simulink library "lib_all"  (Simulink 7.6)
settings: Solver=ode45  SolverMode=SingleTasking  StartTime=0.0  StopTime=10.0  RelTol=1e-3
values from neighbouring files (symbols this model uses):
  map_lib_03 = [6.8679 7.0159 7.4571 8.1849 9.1892 10.456 11.97 13.71 15.655 17.781 20.063 22.474 ... (803 elements, 73x11)]    [scr_simdata.mat]    (also in scr_simdata_all.mat)
  map_lib_06 = [2.5784 2.6727 2.9588 3.4453 4.1444 5.068 6.226 7.6242 9.2634 11.139 13.24 15.552 ... (803 elements, 73x11)]    [scr_simdata.mat]    (also in scr_simdata_all.mat)
  map_lib_09 = [0.0025784 0.0026727 0.0029588 0.0034453 0.0041444 0.005068 0.006226 0.0076242 0.0092634 0.011139 0.01324 0.015552 ... (803 elements, 73x11)]    [scr_simdata.mat]    (also in scr_simdata_all.mat)
  map_lib_10 = [2.74 2.75 2.76 2.77 2.78 2.79 2.8 2.81 2.82 2.83 2.84 2.85 ... (81 elements, 1x81)]    [scr_simdata.mat]    (also in scr_simdata_all.mat)
  map_lib_11 = [1.1983 1.2027 1.207 1.2113 1.2156 1.2198 1.2241 1.2283 1.2326 1.2368 1.241 1.2451 ... (81 elements, 1x81)]    [scr_simdata.mat]    (also in scr_simdata_all.mat)
  map_lib_12 = [2.74 2.75 2.76 2.77 2.78 2.79 2.8 2.81 2.82 2.83 2.84 2.85 ... (81 elements, 1x81)]    [scr_simdata.mat]    (also in scr_simdata_all.mat)
  map_lib_13 = [1.74 1.75 1.76 1.77 1.78 1.79 1.8 1.81 1.82 1.83 1.84 1.85 ... (81 elements, 1x81)]    [scr_simdata.mat]    (also in scr_simdata_all.mat)
  map_lib_16 = [0.00048828125 1.6159 1.8962 2.0821 2.2251 2.3426 2.4433 2.5318 2.611 2.683 2.749 2.8101 ... (1419 elements, 129x11)]    [scr_simdata.mat]    (also in scr_simdata_all.mat)
  map_mr2_01 = [0 800 1600 2400 3200 4000]    [scr_simdata.mat]    (also in scr_simdata_all.mat)
  map_mr2_02 = [1.32 1.32 1.33 1.34 1.36 1.38]    [scr_simdata.mat]    (also in scr_simdata_all.mat)
  map_mr2_03 = [0 800 1600 2400 3200 4000]    [scr_simdata.mat]    (also in scr_simdata_all.mat)
  map_mr2_04 = [1.32 1.32 1.33 1.34 1.36 1.38]    [scr_simdata.mat]    (also in scr_simdata_all.mat)
  map_mr2_05 = [0 800 1600 2400 3200 4000]    [scr_simdata.mat]    (also in scr_simdata_all.mat)
  map_mr2_06 = [1.32 1.32 1.33 1.34 1.36 1.38]    [scr_simdata.mat]    (also in scr_simdata_all.mat)
  Parameter1 = 6    [scr_simdata.mat]    (also in scr_simdata_all.mat)
  Parameter101 = 30    [scr_simdata.mat]    (also in scr_simdata_all.mat)
  Parameter102 = 12    [scr_simdata.mat]    (also in scr_simdata_all.mat)
  Parameter103 = -240    [scr_simdata.mat]    (also in scr_simdata_all.mat)
  Parameter108 = 48    [scr_simdata.mat]    (also in scr_simdata_all.mat)
  Parameter120 = 16    [scr_simdata.mat]    (also in scr_simdata_all.mat)
  Parameter121 = 87    [scr_simdata.mat]    (also in scr_simdata_all.mat)
  Parameter123 = 78    [scr_simdata.mat]    (also in scr_simdata_all.mat)
  Parameter124 = 60    [scr_simdata.mat]    (also in scr_simdata_all.mat)
  Parameter125 = -480    [scr_simdata.mat]    (also in scr_simdata_all.mat)
  Parameter130 = 96    [scr_simdata.mat]    (also in scr_simdata_all.mat)
  Parameter131 = 3    [scr_simdata.mat]    (also in scr_simdata_all.mat)
  Parameter144 = 3    [scr_simdata.mat]    (also in scr_simdata_all.mat)
  Parameter18 = 128    [scr_simdata.mat]    (also in scr_simdata_all.mat)
  Parameter19 = 16    [scr_simdata.mat]    (also in scr_simdata_all.mat)
  Parameter2 = 12    [scr_simdata.mat]    (also in scr_simdata_all.mat)
  Parameter20 = 7    [scr_simdata.mat]    (also in scr_simdata_all.mat)
  Parameter21 = 4    [scr_simdata.mat]    (also in scr_simdata_all.mat)
  Parameter3 = 18    [scr_simdata.mat]    (also in scr_simdata_all.mat)
  Parameter32 = 1    [scr_simdata.mat]    (also in scr_simdata_all.mat)
  Parameter34 = 24    [scr_simdata.mat]    (also in scr_simdata_all.mat)
  Parameter4 = 138    [scr_simdata.mat]    (also in scr_simdata_all.mat)
  Parameter42 = 48    [scr_simdata.mat]    (also in scr_simdata_all.mat)
  Parameter5 = 135    [scr_simdata.mat]    (also in scr_simdata_all.mat)
  Parameter55 = 0    [scr_simdata.mat]    (also in scr_simdata_all.mat)
  Parameter56 = 0    [scr_simdata.mat]    (also in scr_simdata_all.mat)
  Parameter57 = 1    [scr_simdata.mat]    (also in scr_simdata_all.mat)
  Parameter58 = 1    [scr_simdata.mat]    (also in scr_simdata_all.mat)
  Parameter59 = 2    [scr_simdata.mat]    (also in scr_simdata_all.mat)
  Parameter6 = 132    [scr_simdata.mat]    (also in scr_simdata_all.mat)
  Parameter60 = 2    [scr_simdata.mat]    (also in scr_simdata_all.mat)
  Parameter63 = 144    [scr_simdata.mat]    (also in scr_simdata_all.mat)
  Parameter7 = 126    [scr_simdata.mat]    (also in scr_simdata_all.mat)
  Parameter76 = 64    [scr_simdata.mat]    (also in scr_simdata_all.mat)
  Parameter77 = 135    [scr_simdata.mat]    (also in scr_simdata_all.mat)
  Parameter79 = 126    [scr_simdata.mat]    (also in scr_simdata_all.mat)
  Parameter80 = 108    [scr_simdata.mat]    (also in scr_simdata_all.mat)
  Parameter81 = 0    [scr_simdata.mat]    (also in scr_simdata_all.mat)
  Parameter86 = 0    [scr_simdata.mat]    (also in scr_simdata_all.mat)
  Parameter98 = 112    [scr_simdata.mat]    (also in scr_simdata_all.mat)
  Parameter99 = 39    [scr_simdata.mat]    (also in scr_simdata_all.mat)
undefined symbols (not defined in the file or in its folder): cylvolkp_hih_map2h, cylvolkp_map2h, pk1k_map2h
libraries: simulink x2
(part 21 of 36)

== system lib_all/SubSystem347/SubSystem349/SubSystem352/SubSystem466/SubSystem469 ==
  Block1042 : Inport
  Block1043 : Inport  Port=2
  Block1044 : ActionPort
  SubSystem470 : Subsystem  TreatAsAtomicUnit=on  mask(***ATC.DSWVEC***){bd_version=1.01.A, r0=DSM392, r1=-1, r2=, r3=, r4=, r5=, r6=, r7=, r8=, r9=, c0=, c1=, c2=, c3=, c4=, c5=, c6=, c7=, c8=, c9=}
      mask prompts: bd_version "Version"
      mask help: これはAutoCからのブロックです．編集禁止．
      settings: OpenFcn=warndlg('変更禁止!');
      mask Help: web(ui_blockhelpmng(gcb));
      mask Display: disp('ATC.DSRVEC')
  wires:
  Block1043/1 -> SubSystem470/2(element)
  Block1042/1 -> SubSystem470/1(input)

== system lib_all/SubSystem347/SubSystem349/SubSystem352/SubSystem466/SubSystem471 ==
  Block1045 : Inport
  Block1046 : Inport  Port=2
  Block1047 : ActionPort
  SubSystem472 : Subsystem  TreatAsAtomicUnit=on  mask(***ATC.DSWVEC***){bd_version=1.01.A, r0=DSM394, r1=-1, r2=, r3=, r4=, r5=, r6=, r7=, r8=, r9=, c0=, c1=, c2=, c3=, c4=, c5=, c6=, c7=, c8=, c9=}
      mask prompts: bd_version "Version"
      mask help: これはAutoCからのブロックです．編集禁止．
      settings: OpenFcn=warndlg('変更禁止!');
      mask Help: web(ui_blockhelpmng(gcb));
      mask Display: disp('ATC.DSRVEC')
  wires:
  Block1046/1 -> SubSystem472/2(element)
  Block1045/1 -> SubSystem472/1(input)

== system lib_all/SubSystem347/SubSystem349/SubSystem352/SubSystem473/SubSystem474 ==
  note: ***編集禁止***
  element : Inport
  Inside_DSR : DataStoreRead  DataStoreName=DSM386
  Selector : Selector  IndexOptions=Index vector (port)  Indices=[1 3]  OutputSizes=1
  Output : Outport
  wires:
  Selector/1 -> Output/1
  element/1 -> Selector/2
  Inside_DSR/1 -> Selector/1

== system lib_all/SubSystem347/SubSystem349/SubSystem352/SubSystem473/SubSystem475 ==
  note: ***編集禁止***
  input : Inport
  element : Inport  Port=2
  Assignment : Assignment  IndexOptions=Index vector (port)  Indices=1  OutputSizes=[1 1]
  Inside_DSR : DataStoreRead  DataStoreName=DSM386
  Inside_DSW : DataStoreWrite  DataStoreName=DSM386
  wires:
  element/1 -> Assignment/3
  input/1 -> Assignment/2
  Inside_DSR/1 -> Assignment/1
  Assignment/1 -> Inside_DSW/1

== system lib_all/SubSystem347/SubSystem477/SubSystem479/SubSystem482/SubSystem483 ==
  note: ***編集禁止***
  In1 : Inport
  In2 : Inport  Port=2
  RO1 : RelationalOperator  Operator=<=  InputSameDT=on
  single1 : DataTypeConversion  OutDataTypeStr=single  SaturateOnIntegerOverflow=on  RndMeth=Zero
  single2 : DataTypeConversion  OutDataTypeStr=single  SaturateOnIntegerOverflow=on  RndMeth=Zero
  Out1 : Outport
  wires:
  RO1/1 -> Out1/1
  In2/1 -> single2/1
  In1/1 -> single1/1
  single1/1 -> RO1/1
  single2/1 -> RO1/2

== system lib_all/SubSystem347/SubSystem477/SubSystem479/SubSystem482/SubSystem484 ==
  note: ***編集禁止***
  input : Inport
  element : Inport  Port=2
  Assignment : Assignment  IndexOptions=Index vector (port)  Indices=1  OutputSizes=[1 1]
  Inside_DSR : DataStoreRead  DataStoreName=DSM383
  Inside_DSW : DataStoreWrite  DataStoreName=DSM383
  wires:
  element/1 -> Assignment/3
  input/1 -> Assignment/2
  Inside_DSR/1 -> Assignment/1
  Assignment/1 -> Inside_DSW/1

== system lib_all/SubSystem347/SubSystem477/SubSystem479/SubSystem482/SubSystem485 ==
  note: ***編集禁止***
  input : Inport
  element : Inport  Port=2
  Assignment : Assignment  IndexOptions=Index vector (port)  Indices=1  OutputSizes=[1 1]
  Inside_DSR : DataStoreRead  DataStoreName=DSM385
  Inside_DSW : DataStoreWrite  DataStoreName=DSM385
  wires:
  element/1 -> Assignment/3
  input/1 -> Assignment/2
  Inside_DSR/1 -> Assignment/1
  Assignment/1 -> Inside_DSW/1

== system lib_all/SubSystem347/SubSystem477/SubSystem479/SubSystem482/SubSystem486 ==
  note: ***編集禁止***
  input : Inport
  element : Inport  Port=2
  Assignment : Assignment  IndexOptions=Index vector (port)  Indices=1  OutputSizes=[1 1]
  Inside_DSR : DataStoreRead  DataStoreName=DSM387
  Inside_DSW : DataStoreWrite  DataStoreName=DSM387
  wires:
  element/1 -> Assignment/3
  input/1 -> Assignment/2
  Inside_DSR/1 -> Assignment/1
  Assignment/1 -> Inside_DSW/1

== system lib_all/SubSystem347/SubSystem477/SubSystem479/SubSystem482/SubSystem487 ==
  Block1085 : EnablePort
  Block1086 : Constant  Value=Parameter131  OutDataTypeStr=double  SampleTime=-1
  Block1087 : Constant  Value=10  OutDataTypeStr=double  SampleTime=-1
  Block1088 : DataStoreWrite  DataStoreName=DSM365
  SubSystem488 : Subsystem  TreatAsAtomicUnit=on
  SubSystem490 : Subsystem  TreatAsAtomicUnit=on
      settings: RTWSystemCode=Inline
  wires:
  Block1087/1 -> Block1088/1
  Block1086/1 -> SubSystem490/1(N)
  SubSystem490/1(action) -> SubSystem488/Trigger
  SubSystem490/2(i) -> SubSystem488/1(Block1089)

== system lib_all/SubSystem347/SubSystem477/SubSystem479/SubSystem492/SubSystem493 ==
  note: ***編集禁止***
  In1 : Inport
  In2 : Inport  Port=2
  RO1 : RelationalOperator  Operator===  InputSameDT=on
  single1 : DataTypeConversion  OutDataTypeStr=single  SaturateOnIntegerOverflow=on  RndMeth=Zero
  single2 : DataTypeConversion  OutDataTypeStr=single  SaturateOnIntegerOverflow=on  RndMeth=Zero
  Out1 : Outport
  wires:
  RO1/1 -> Out1/1
  In2/1 -> single2/1
  In1/1 -> single1/1
  single1/1 -> RO1/1
  single2/1 -> RO1/2

== system lib_all/SubSystem347/SubSystem477/SubSystem479/SubSystem492/SubSystem494 ==
  Block1105 : Inport
  Block1106 : Inport  Port=2
  Block1107 : MultiPortSwitch  zeroidx=off  RndMeth=Zero  SaturateOnIntegerOverflow=on  InputSameDT=on
  SubSystem495 : Subsystem  TreatAsAtomicUnit=on  mask(***ATC.DSRVEC***){bd_version=1.01.A, r0=DSM382, r1=-1, r2=, r3=, r4=, r5=, r6=, r7=, r8=, r9=, c0=, c1=, c2=, c3=, c4=, c5=, c6=, c7=, c8=, c9=}
      mask prompts: bd_version "Version"
      mask help: これはAutoCからのブロックです．編集禁止．
      settings: OpenFcn=warndlg('変更禁止!');
      mask Help: web(ui_blockhelpmng(gcb));
      mask Display: disp('ATC.DSRVEC')
  SubSystem496 : Subsystem  TreatAsAtomicUnit=on  mask(***ATC.DSRVEC***){bd_version=1.01.A, r0=DSM391, r1=-1, r2=, r3=, r4=, r5=, r6=, r7=, r8=, r9=, c0=, c1=, c2=, c3=, c4=, c5=, c6=, c7=, c8=, c9=}
      mask prompts: bd_version "Version"
      mask help: これはAutoCからのブロックです．編集禁止．
      settings: OpenFcn=warndlg('変更禁止!');
      mask Help: web(ui_blockhelpmng(gcb));
      mask Display: disp('ATC.DSRVEC')
  SubSystem497 : Subsystem  TreatAsAtomicUnit=on  mask(***ATC.DSRVEC***){bd_version=1.01.A, r0=DSM393, r1=-1, r2=, r3=, r4=, r5=, r6=, r7=, r8=, r9=, c0=, c1=, c2=, c3=, c4=, c5=, c6=, c7=, c8=, c9=}
      mask prompts: bd_version "Version"
      mask help: これはAutoCからのブロックです．編集禁止．
      settings: OpenFcn=warndlg('変更禁止!');
      mask Help: web(ui_blockhelpmng(gcb));
      mask Display: disp('ATC.DSRVEC')
  Block1108 : Outport
  wires:
  Block1106/1 -> SubSystem497/1(element), SubSystem496/1(element), SubSystem495/1(element)
  Block1107/1 -> Block1108/1
  SubSystem495/1(Output) -> Block1107/2
  SubSystem496/1(Output) -> Block1107/3
  SubSystem497/1(Output) -> Block1107/4
  Block1105/1 -> Block1107/1

== system lib_all/SubSystem347/SubSystem477/SubSystem479/SubSystem492/SubSystem498 ==
  Block1109 : Inport
  Block1110 : Inport  Port=2
  Block1111 : MultiPortSwitch  zeroidx=off  RndMeth=Zero  SaturateOnIntegerOverflow=on  InputSameDT=on
  SubSystem499 : Subsystem  TreatAsAtomicUnit=on  mask(***ATC.DSRVEC***){bd_version=1.01.A, r0=DSM384, r1=-1, r2=, r3=, r4=, r5=, r6=, r7=, r8=, r9=, c0=, c1=, c2=, c3=, c4=, c5=, c6=, c7=, c8=, c9=}
      mask prompts: bd_version "Version"
      mask help: これはAutoCからのブロックです．編集禁止．
      settings: OpenFcn=warndlg('変更禁止!');
      mask Help: web(ui_blockhelpmng(gcb));
      mask Display: disp('ATC.DSRVEC')
  SubSystem500 : Subsystem  TreatAsAtomicUnit=on  mask(***ATC.DSRVEC***){bd_version=1.01.A, r0=DSM392, r1=-1, r2=, r3=, r4=, r5=, r6=, r7=, r8=, r9=, c0=, c1=, c2=, c3=, c4=, c5=, c6=, c7=, c8=, c9=}
      mask prompts: bd_version "Version"
      mask help: これはAutoCからのブロックです．編集禁止．
      settings: OpenFcn=warndlg('変更禁止!');
      mask Help: web(ui_blockhelpmng(gcb));
      mask Display: disp('ATC.DSRVEC')
  SubSystem501 : Subsystem  TreatAsAtomicUnit=on  mask(***ATC.DSRVEC***){bd_version=1.01.A, r0=DSM394, r1=-1, r2=, r3=, r4=, r5=, r6=, r7=, r8=, r9=, c0=, c1=, c2=, c3=, c4=, c5=, c6=, c7=, c8=, c9=}
      mask prompts: bd_version "Version"
      mask help: これはAutoCからのブロックです．編集禁止．
      settings: OpenFcn=warndlg('変更禁止!');
      mask Help: web(ui_blockhelpmng(gcb));
      mask Display: disp('ATC.DSRVEC')
  Block1112 : Outport
  wires:
  Block1110/1 -> SubSystem501/1(element), SubSystem500/1(element), SubSystem499/1(element)
  SubSystem501/1(Output) -> Block1111/4
  SubSystem500/1(Output) -> Block1111/3
  SubSystem499/1(Output) -> Block1111/2
  Block1111/1 -> Block1112/1
  Block1109/1 -> Block1111/1

== system lib_all/SubSystem347/SubSystem503/SubSystem506/SubSystem511/SubSystem512 ==
  note: ***編集禁止***
  input : Inport
  element : Inport  Port=2
  Assignment : Assignment  IndexOptions=Index vector (port)  Indices=1  OutputSizes=[1 1]
  Inside_DSR : DataStoreRead  DataStoreName=DSM87
  Inside_DSW : DataStoreWrite  DataStoreName=DSM87
  wires:
  element/1 -> Assignment/3
  input/1 -> Assignment/2
  Inside_DSR/1 -> Assignment/1
  Assignment/1 -> Inside_DSW/1

== system lib_all/SubSystem347/SubSystem503/SubSystem506/SubSystem511/SubSystem513 ==
  note: ***編集禁止***
  element : Inport
  Inside_DSR : DataStoreRead  DataStoreName=DSM387
  Selector : Selector  IndexOptions=Index vector (port)  Indices=[1 3]  OutputSizes=1
  Output : Outport
  wires:
  Selector/1 -> Output/1
  element/1 -> Selector/2
  Inside_DSR/1 -> Selector/1

== system lib_all/SubSystem347/SubSystem503/SubSystem506/SubSystem511/SubSystem514 ==
  note: ***編集禁止***
  element : Inport
  Inside_DSR : DataStoreRead  DataStoreName=DSM387
  Selector : Selector  IndexOptions=Index vector (port)  Indices=[1 3]  OutputSizes=1
  Output : Outport
  wires:
  Selector/1 -> Output/1
  element/1 -> Selector/2
  Inside_DSR/1 -> Selector/1

== system lib_all/SubSystem347/SubSystem503/SubSystem506/SubSystem515/SubSystem516 ==
  note: ***編集禁止***
  input : Inport
  element : Inport  Port=2
  Assignment : Assignment  IndexOptions=Index vector (port)  Indices=1  OutputSizes=[1 1]
  Inside_DSR : DataStoreRead  DataStoreName=DSM386
  Inside_DSW : DataStoreWrite  DataStoreName=DSM386
  wires:
  element/1 -> Assignment/3
  input/1 -> Assignment/2
  Inside_DSR/1 -> Assignment/1
  Assignment/1 -> Inside_DSW/1

== system lib_all/SubSystem518/SubSystem523/SubSystem524/SubSystem527/SubSystem528 ==
  note: ***編集禁止***
  mult : Inport
  div : Inport  Port=2
  init : Inport  Port=3
  Product : Subsystem  TreatAsAtomicUnit=on
      settings: RTWSystemCode=Inline
  Out : Outport
  wires:
  init/1 -> Product/3(init)
  div/1 -> Product/2(div)
  mult/1 -> Product/1(mult)
  Product/1(Out) -> Out/1

== system lib_all/SubSystem518/SubSystem523/SubSystem524/SubSystem527/SubSystem529 ==
  note: ***編集禁止***
  element : Inport
  Inside_DSR : DataStoreRead  DataStoreName=DSM95
  Selector : Selector  IndexOptions=Index vector (port)  Indices=[1 3]  OutputSizes=1
  Output : Outport
  wires:
  Inside_DSR/1 -> Selector/1
  element/1 -> Selector/2
  Selector/1 -> Output/1

== system lib_all/SubSystem518/SubSystem523/SubSystem524/SubSystem527/SubSystem530 ==
  Block1193 : Inport
  Block1194 : Inport  Port=2
  Block1195 : Inport  Port=3
  Block1196 : Sum  OutDataTypeStr=double  RndMeth=Zero  SaturateOnIntegerOverflow=on  InputSameDT=on
  Block1197 : Sum  Inputs=+-  OutDataTypeStr=double  RndMeth=Zero  SaturateOnIntegerOverflow=on  InputSameDT=on
  Block1198 : Switch  Threshold=0.5  RndMeth=Zero  SaturateOnIntegerOverflow=on  InputSameDT=on
  Block1199 : Switch  Threshold=0.5  RndMeth=Zero  SaturateOnIntegerOverflow=on  InputSameDT=on
  SubSystem531 : Subsystem
  SubSystem532 : Subsystem
  Block1200 : Outport
  wires:
  Block1197/1 -> Block1198/1
  Block1198/1 -> Block1200/1
  Block1196/1 -> Block1199/1
  Block1199/1 -> Block1198/3
  SubSystem532/1(Out1) -> Block1199/2
  Block1195/1 -> SubSystem532/2(In2)
  SubSystem531/1(Out1) -> Block1198/2
  Block1193/1 -> SubSystem531/2(In2), Block1197/2, Block1196/1
  Block1194/1 -> Block1196/2, Block1199/3, SubSystem532/1(In1), Block1197/1, SubSystem531/1(In1)

== system lib_all/SubSystem518/SubSystem523/SubSystem524/SubSystem533/SubSystem534 ==
  note: ***編集禁止***
  In1 : Inport
  In2 : Inport  Port=2
  LO1 : Logic
  RO1 : RelationalOperator  Operator=<  InputSameDT=on
  RO2 : RelationalOperator  Operator=~=  InputSameDT=on
  single1 : DataTypeConversion  OutDataTypeStr=single  SaturateOnIntegerOverflow=on  RndMeth=Zero
  single2 : DataTypeConversion  OutDataTypeStr=single  SaturateOnIntegerOverflow=on  RndMeth=Zero
  Out1 : Outport
  wires:
  single2/1 -> RO1/2, RO2/2
  single1/1 -> RO1/1, RO2/1
  In1/1 -> single1/1
  In2/1 -> single2/1
  RO1/1 -> LO1/1
  RO2/1 -> LO1/2
  LO1/1 -> Out1/1

== system lib_all/SubSystem518/SubSystem523/SubSystem524/SubSystem533/SubSystem535 ==
  note: ***編集禁止***
  In1 : Inport
  In2 : Inport  Port=2
  RO1 : RelationalOperator  Operator===  InputSameDT=on
  single1 : DataTypeConversion  OutDataTypeStr=single  SaturateOnIntegerOverflow=on  RndMeth=Zero
  single2 : DataTypeConversion  OutDataTypeStr=single  SaturateOnIntegerOverflow=on  RndMeth=Zero
  Out1 : Outport
  wires:
  single2/1 -> RO1/2
  single1/1 -> RO1/1
  In1/1 -> single1/1
  In2/1 -> single2/1
  RO1/1 -> Out1/1

== system lib_all/SubSystem518/SubSystem523/SubSystem524/SubSystem533/SubSystem536 ==
  note: ***編集禁止***
  In1 : Inport
  In2 : Inport  Port=2
  RO1 : RelationalOperator  Operator===  InputSameDT=on
  single1 : DataTypeConversion  OutDataTypeStr=single  SaturateOnIntegerOverflow=on  RndMeth=Zero
  single2 : DataTypeConversion  OutDataTypeStr=single  SaturateOnIntegerOverflow=on  RndMeth=Zero
  Out1 : Outport
  wires:
  single2/1 -> RO1/2
  single1/1 -> RO1/1
  In1/1 -> single1/1
  In2/1 -> single2/1
  RO1/1 -> Out1/1

== system lib_all/SubSystem518/SubSystem523/SubSystem524/SubSystem533/SubSystem537 ==
  note: ***編集禁止***
  In1 : Inport
  In2 : Inport  Port=2
  RO1 : RelationalOperator  Operator===  InputSameDT=on
  single1 : DataTypeConversion  OutDataTypeStr=single  SaturateOnIntegerOverflow=on  RndMeth=Zero
  single2 : DataTypeConversion  OutDataTypeStr=single  SaturateOnIntegerOverflow=on  RndMeth=Zero
  Out1 : Outport
  wires:
  single2/1 -> RO1/2
  single1/1 -> RO1/1
  In1/1 -> single1/1
  In2/1 -> single2/1
  RO1/1 -> Out1/1

== system lib_all/SubSystem518/SubSystem523/SubSystem524/SubSystem533/SubSystem538 ==
  Block1223 : Inport
  Block1224 : Inport  Port=2
  Block1225 : EnablePort
  Block1226 : Demux  Outputs=2
  Block1227 : Mux  Inputs=2
  SubSystem539 : Subsystem
  SubSystem543 : Subsystem
  Block1236 : Outport
  wires:
  Block1227/1 -> Block1236/1
  SubSystem543/1(Block1235) -> Block1227/2
  SubSystem539/1(Block1231) -> Block1227/1
  Block1226/2 -> SubSystem543/2(Block1233)
  Block1226/1 -> SubSystem539/2(Block1229)
  Block1224/1 -> SubSystem539/1(Block1228), SubSystem543/1(Block1232)
  Block1223/1 -> Block1226/1

== system lib_all/SubSystem518/SubSystem523/SubSystem524/SubSystem533/SubSystem547 ==
  Block1237 : Inport
  Block1238 : Inport  Port=2
  Block1239 : EnablePort
  Block1240 : Demux  Outputs=2
  Block1241 : Mux  Inputs=2
  Block1242 : Sum  OutDataTypeStr=double  RndMeth=Zero  SaturateOnIntegerOverflow=on  InputSameDT=on
  SubSystem548 : Subsystem  TreatAsAtomicUnit=on  mask(***ATC.DSRVEC***){bd_version=1.01.A, r0=DSM1, r1=-1, r2=, r3=, r4=, r5=, r6=, r7=, r8=, r9=, c0=, c1=, c2=, c3=, c4=, c5=, c6=, c7=, c8=, c9=}
      mask prompts: bd_version "Version"
      mask help: これはAutoCからのブロックです．編集禁止．
      settings: OpenFcn=warndlg('変更禁止!');
      mask Help: web(ui_blockhelpmng(gcb));
      mask Display: disp('ATC.DSRVEC')
  SubSystem549 : Subsystem
  SubSystem553 : Subsystem
  Block1251 : Outport
  wires:
  Block1238/1 -> SubSystem548/1(element), SubSystem549/1(Block1243), SubSystem553/1(Block1247)
  Block1241/1 -> Block1242/2
  SubSystem548/1(Output) -> Block1242/1
  Block1242/1 -> Block1251/1
  SubSystem553/1(Block1250) -> Block1241/2
  SubSystem549/1(Block1246) -> Block1241/1
  Block1240/2 -> SubSystem553/2(Block1248)
  Block1240/1 -> SubSystem549/2(Block1244)
  Block1237/1 -> Block1240/1

== system lib_all/SubSystem518/SubSystem523/SubSystem524/SubSystem533/SubSystem557 ==
  Block1252 : Inport
  Block1253 : Inport  Port=2
  Block1254 : EnablePort
  Block1255 : Demux  Outputs=2
  Block1256 : Mux  Inputs=2
  Block1257 : Sum  OutDataTypeStr=double  RndMeth=Zero  SaturateOnIntegerOverflow=on  InputSameDT=on
  SubSystem558 : Subsystem  TreatAsAtomicUnit=on  mask(***ATC.DSRVEC***){bd_version=1.01.A, r0=DSM5, r1=-1, r2=, r3=, r4=, r5=, r6=, r7=, r8=, r9=, c0=, c1=, c2=, c3=, c4=, c5=, c6=, c7=, c8=, c9=}
      mask prompts: bd_version "Version"
      mask help: これはAutoCからのブロックです．編集禁止．
      settings: OpenFcn=warndlg('変更禁止!');
      mask Help: web(ui_blockhelpmng(gcb));
      mask Display: disp('ATC.DSRVEC')
  SubSystem559 : Subsystem
  SubSystem563 : Subsystem
  Block1266 : Outport
  wires:
  Block1253/1 -> SubSystem558/1(element), SubSystem559/1(Block1258), SubSystem563/1(Block1262)
  Block1256/1 -> Block1257/2
  SubSystem558/1(Output) -> Block1257/1
  Block1257/1 -> Block1266/1
  SubSystem563/1(Block1265) -> Block1256/2
  SubSystem559/1(Block1261) -> Block1256/1
  Block1255/2 -> SubSystem563/2(Block1263)
  Block1255/1 -> SubSystem559/2(Block1259)
  Block1252/1 -> Block1255/1

== system lib_all/SubSystem518/SubSystem523/SubSystem524/SubSystem533/SubSystem567 ==
  Block1267 : Inport
  Block1268 : Inport  Port=2
  Block1269 : EnablePort
  Block1270 : Demux  Outputs=2
  Block1271 : Mux  Inputs=2
  Block1272 : Sum  OutDataTypeStr=double  RndMeth=Zero  SaturateOnIntegerOverflow=on  InputSameDT=on
  SubSystem568 : Subsystem  TreatAsAtomicUnit=on  mask(***ATC.DSRVEC***){bd_version=1.01.A, r0=DSM3, r1=-1, r2=, r3=, r4=, r5=, r6=, r7=, r8=, r9=, c0=, c1=, c2=, c3=, c4=, c5=, c6=, c7=, c8=, c9=}
      mask prompts: bd_version "Version"
      mask help: これはAutoCからのブロックです．編集禁止．
      settings: OpenFcn=warndlg('変更禁止!');
      mask Help: web(ui_blockhelpmng(gcb));
      mask Display: disp('ATC.DSRVEC')
  SubSystem569 : Subsystem
  SubSystem573 : Subsystem
  Block1281 : Outport
  wires:
  Block1268/1 -> SubSystem568/1(element), SubSystem569/1(Block1273), SubSystem573/1(Block1277)
  Block1267/1 -> Block1270/1
  Block1270/1 -> SubSystem569/2(Block1274)
  Block1270/2 -> SubSystem573/2(Block1278)
  SubSystem569/1(Block1276) -> Block1271/1
  SubSystem573/1(Block1280) -> Block1271/2
  Block1272/1 -> Block1281/1
  SubSystem568/1(Output) -> Block1272/1
  Block1271/1 -> Block1272/2

== system lib_all/SubSystem518/SubSystem523/SubSystem577/SubSystem580/SubSystem581 ==
  note: ***編集禁止***
  mult : Inport
  div : Inport  Port=2
  init : Inport  Port=3
  Product : Subsystem  TreatAsAtomicUnit=on
      settings: RTWSystemCode=Inline
  Out : Outport
  wires:
  init/1 -> Product/3(init)
  div/1 -> Product/2(div)
  mult/1 -> Product/1(mult)
  Product/1(Out) -> Out/1

== system lib_all/SubSystem518/SubSystem523/SubSystem577/SubSystem580/SubSystem582 ==
  note: ***編集禁止***
  element : Inport
  Inside_DSR : DataStoreRead  DataStoreName=DSM95
  Selector : Selector  IndexOptions=Index vector (port)  Indices=[1 3]  OutputSizes=1
  Output : Outport
  wires:
  Inside_DSR/1 -> Selector/1
  element/1 -> Selector/2
  Selector/1 -> Output/1

== system lib_all/SubSystem518/SubSystem523/SubSystem577/SubSystem580/SubSystem583 ==
  Block1315 : Inport
  Block1316 : Inport  Port=2
  Block1317 : Inport  Port=3
  Block1318 : Sum  OutDataTypeStr=double  RndMeth=Zero  SaturateOnIntegerOverflow=on  InputSameDT=on
  Block1319 : Sum  Inputs=+-  OutDataTypeStr=double  RndMeth=Zero  SaturateOnIntegerOverflow=on  InputSameDT=on
  Block1320 : Switch  Threshold=0.5  RndMeth=Zero  SaturateOnIntegerOverflow=on  InputSameDT=on
  Block1321 : Switch  Threshold=0.5  RndMeth=Zero  SaturateOnIntegerOverflow=on  InputSameDT=on
  SubSystem584 : Subsystem
  SubSystem585 : Subsystem
  Block1322 : Outport
  wires:
  Block1319/1 -> Block1320/1
  Block1320/1 -> Block1322/1
  Block1318/1 -> Block1321/1
  Block1321/1 -> Block1320/3
  SubSystem585/1(Out1) -> Block1321/2
  Block1317/1 -> SubSystem585/2(In2)
  SubSystem584/1(Out1) -> Block1320/2
  Block1315/1 -> SubSystem584/2(In2), Block1319/2, Block1318/1
  Block1316/1 -> Block1318/2, Block1321/3, SubSystem585/1(In1), Block1319/1, SubSystem584/1(In1)

== system lib_all/SubSystem518/SubSystem523/SubSystem577/SubSystem586/SubSystem587 ==
  note: ***編集禁止***
  In1 : Inport
  In2 : Inport  Port=2
  LO1 : Logic
  RO1 : RelationalOperator  Operator=<  InputSameDT=on
  RO2 : RelationalOperator  Operator=~=  InputSameDT=on
  single1 : DataTypeConversion  OutDataTypeStr=single  SaturateOnIntegerOverflow=on  RndMeth=Zero
  single2 : DataTypeConversion  OutDataTypeStr=single  SaturateOnIntegerOverflow=on  RndMeth=Zero
  Out1 : Outport
  wires:
  single2/1 -> RO1/2, RO2/2
  single1/1 -> RO1/1, RO2/1
  In1/1 -> single1/1
  In2/1 -> single2/1
  RO1/1 -> LO1/1
  RO2/1 -> LO1/2
  LO1/1 -> Out1/1

== system lib_all/SubSystem518/SubSystem523/SubSystem577/SubSystem586/SubSystem588 ==
  note: ***編集禁止***
  In1 : Inport
  In2 : Inport  Port=2
  RO1 : RelationalOperator  Operator===  InputSameDT=on
  single1 : DataTypeConversion  OutDataTypeStr=single  SaturateOnIntegerOverflow=on  RndMeth=Zero
  single2 : DataTypeConversion  OutDataTypeStr=single  SaturateOnIntegerOverflow=on  RndMeth=Zero
  Out1 : Outport
  wires:
  single2/1 -> RO1/2
  single1/1 -> RO1/1
  In1/1 -> single1/1
  In2/1 -> single2/1
  RO1/1 -> Out1/1

== system lib_all/SubSystem518/SubSystem523/SubSystem577/SubSystem586/SubSystem589 ==
  note: ***編集禁止***
  In1 : Inport
  In2 : Inport  Port=2
  RO1 : RelationalOperator  Operator===  InputSameDT=on
  single1 : DataTypeConversion  OutDataTypeStr=single  SaturateOnIntegerOverflow=on  RndMeth=Zero
  single2 : DataTypeConversion  OutDataTypeStr=single  SaturateOnIntegerOverflow=on  RndMeth=Zero
  Out1 : Outport
  wires:
  single2/1 -> RO1/2
  single1/1 -> RO1/1
  In1/1 -> single1/1
  In2/1 -> single2/1
  RO1/1 -> Out1/1

== system lib_all/SubSystem518/SubSystem523/SubSystem577/SubSystem586/SubSystem590 ==
  note: ***編集禁止***
  In1 : Inport
  In2 : Inport  Port=2
  RO1 : RelationalOperator  Operator===  InputSameDT=on
  single1 : DataTypeConversion  OutDataTypeStr=single  SaturateOnIntegerOverflow=on  RndMeth=Zero
  single2 : DataTypeConversion  OutDataTypeStr=single  SaturateOnIntegerOverflow=on  RndMeth=Zero
  Out1 : Outport
  wires:
  single2/1 -> RO1/2
  single1/1 -> RO1/1
  In1/1 -> single1/1
  In2/1 -> single2/1
  RO1/1 -> Out1/1

== system lib_all/SubSystem518/SubSystem523/SubSystem577/SubSystem586/SubSystem591 ==
  Block1345 : Inport
  Block1346 : Inport  Port=2
  Block1347 : EnablePort
  Block1348 : Demux  Outputs=2
  Block1349 : Mux  Inputs=2
  SubSystem592 : Subsystem
  SubSystem596 : Subsystem
  Block1358 : Outport
  wires:
  Block1349/1 -> Block1358/1
  SubSystem596/1(Block1357) -> Block1349/2
  SubSystem592/1(Block1353) -> Block1349/1
  Block1348/2 -> SubSystem596/2(Block1355)
  Block1348/1 -> SubSystem592/2(Block1351)
  Block1346/1 -> SubSystem592/1(Block1350), SubSystem596/1(Block1354)
  Block1345/1 -> Block1348/1

== system lib_all/SubSystem518/SubSystem523/SubSystem577/SubSystem586/SubSystem600 ==
  Block1359 : Inport
  Block1360 : Inport  Port=2
  Block1361 : EnablePort
  Block1362 : Demux  Outputs=2
  Block1363 : Mux  Inputs=2
  Block1364 : Sum  OutDataTypeStr=double  RndMeth=Zero  SaturateOnIntegerOverflow=on  InputSameDT=on
  SubSystem601 : Subsystem  TreatAsAtomicUnit=on  mask(***ATC.DSRVEC***){bd_version=1.01.A, r0=DSM1, r1=-1, r2=, r3=, r4=, r5=, r6=, r7=, r8=, r9=, c0=, c1=, c2=, c3=, c4=, c5=, c6=, c7=, c8=, c9=}
      mask prompts: bd_version "Version"
      mask help: これはAutoCからのブロックです．編集禁止．
      settings: OpenFcn=warndlg('変更禁止!');
      mask Help: web(ui_blockhelpmng(gcb));
      mask Display: disp('ATC.DSRVEC')
  SubSystem602 : Subsystem
  SubSystem606 : Subsystem
  Block1373 : Outport
  wires:
  Block1360/1 -> SubSystem601/1(element), SubSystem602/1(Block1365), SubSystem606/1(Block1369)
  Block1363/1 -> Block1364/2
  SubSystem601/1(Output) -> Block1364/1
  Block1364/1 -> Block1373/1
  SubSystem606/1(Block1372) -> Block1363/2
  SubSystem602/1(Block1368) -> Block1363/1
  Block1362/2 -> SubSystem606/2(Block1370)
  Block1362/1 -> SubSystem602/2(Block1366)
  Block1359/1 -> Block1362/1

== system lib_all/SubSystem518/SubSystem523/SubSystem577/SubSystem586/SubSystem610 ==
  Block1374 : Inport
  Block1375 : Inport  Port=2
  Block1376 : EnablePort
  Block1377 : Demux  Outputs=2
  Block1378 : Mux  Inputs=2
  Block1379 : Sum  OutDataTypeStr=double  RndMeth=Zero  SaturateOnIntegerOverflow=on  InputSameDT=on
  SubSystem611 : Subsystem  TreatAsAtomicUnit=on  mask(***ATC.DSRVEC***){bd_version=1.01.A, r0=DSM5, r1=-1, r2=, r3=, r4=, r5=, r6=, r7=, r8=, r9=, c0=, c1=, c2=, c3=, c4=, c5=, c6=, c7=, c8=, c9=}
      mask prompts: bd_version "Version"
      mask help: これはAutoCからのブロックです．編集禁止．
      settings: OpenFcn=warndlg('変更禁止!');
      mask Help: web(ui_blockhelpmng(gcb));
      mask Display: disp('ATC.DSRVEC')
  SubSystem612 : Subsystem
  SubSystem616 : Subsystem
  Block1388 : Outport
  wires:
  Block1375/1 -> SubSystem611/1(element), SubSystem612/1(Block1380), SubSystem616/1(Block1384)
  Block1378/1 -> Block1379/2
  SubSystem611/1(Output) -> Block1379/1
  Block1379/1 -> Block1388/1
  SubSystem616/1(Block1387) -> Block1378/2
  SubSystem612/1(Block1383) -> Block1378/1
  Block1377/2 -> SubSystem616/2(Block1385)
  Block1377/1 -> SubSystem612/2(Block1381)
  Block1374/1 -> Block1377/1

== system lib_all/SubSystem518/SubSystem523/SubSystem577/SubSystem586/SubSystem620 ==
  Block1389 : Inport
  Block1390 : Inport  Port=2
  Block1391 : EnablePort
  Block1392 : Demux  Outputs=2
  Block1393 : Mux  Inputs=2
  Block1394 : Sum  OutDataTypeStr=double  RndMeth=Zero  SaturateOnIntegerOverflow=on  InputSameDT=on
  SubSystem621 : Subsystem  TreatAsAtomicUnit=on  mask(***ATC.DSRVEC***){bd_version=1.01.A, r0=DSM3, r1=-1, r2=, r3=, r4=, r5=, r6=, r7=, r8=, r9=, c0=, c1=, c2=, c3=, c4=, c5=, c6=, c7=, c8=, c9=}
      mask prompts: bd_version "Version"
      mask help: これはAutoCからのブロックです．編集禁止．
      settings: OpenFcn=warndlg('変更禁止!');
      mask Help: web(ui_blockhelpmng(gcb));
      mask Display: disp('ATC.DSRVEC')
  SubSystem622 : Subsystem
  SubSystem626 : Subsystem
  Block1403 : Outport
  wires:
  Block1390/1 -> SubSystem621/1(element), SubSystem622/1(Block1395), SubSystem626/1(Block1399)
  Block1389/1 -> Block1392/1
  Block1392/1 -> SubSystem622/2(Block1396)
  Block1392/2 -> SubSystem626/2(Block1400)
  SubSystem622/1(Block1398) -> Block1393/1
  SubSystem626/1(Block1402) -> Block1393/2
  Block1394/1 -> Block1403/1
  SubSystem621/1(Output) -> Block1394/1
  Block1393/1 -> Block1394/2

== system lib_all/SubSystem660/SubSystem673/SubSystem676/SubSystem679/SubSystem680 ==
  note: ***編集禁止***
  In1 : Inport
  In2 : Inport  Port=2
  LO1 : Logic
  RO1 : RelationalOperator  Operator=<  InputSameDT=on
  RO2 : RelationalOperator  Operator=~=  InputSameDT=on
  single1 : DataTypeConversion  OutDataTypeStr=single  SaturateOnIntegerOverflow=on  RndMeth=Zero
  single2 : DataTypeConversion  OutDataTypeStr=single  SaturateOnIntegerOverflow=on  RndMeth=Zero
  Out1 : Outport
  wires:
  LO1/1 -> Out1/1
  RO2/1 -> LO1/2
  RO1/1 -> LO1/1
  single2/1 -> RO2/2, RO1/2
  single1/1 -> RO2/1, RO1/1
  In1/1 -> single1/1
  In2/1 -> single2/1

== system lib_all/SubSystem660/SubSystem673/SubSystem676/SubSystem681/SubSystem682 ==
  note: ***編集禁止***
  In1 : Inport
  In2 : Inport  Port=2
  LO1 : Logic
  RO1 : RelationalOperator  Operator=<  InputSameDT=on
  RO2 : RelationalOperator  Operator=~=  InputSameDT=on
  single1 : DataTypeConversion  OutDataTypeStr=single  SaturateOnIntegerOverflow=on  RndMeth=Zero
  single2 : DataTypeConversion  OutDataTypeStr=single  SaturateOnIntegerOverflow=on  RndMeth=Zero
  Out1 : Outport
  wires:
  LO1/1 -> Out1/1
  RO2/1 -> LO1/2
  RO1/1 -> LO1/1
  single2/1 -> RO2/2, RO1/2
  single1/1 -> RO2/1, RO1/1
  In1/1 -> single1/1
  In2/1 -> single2/1

== system lib_all/SubSystem660/SubSystem686/SubSystem688/SubSystem689/Product ==
  note: ***編集禁止***
  mult : Inport
  div : Inport  Port=2
  init : Inport  Port=3
  Constant1 : Constant  OutDataTypeStr=Inherit: Inherit via back propagation
  Constant2 : Constant  Value=0  OutDataTypeStr=Inherit: Inherit via back propagation
  Product : Product  Inputs=*/  SaturateOnIntegerOverflow=on  RndMeth=Zero  InputSameDT=on  OutDataTypeStr=Inherit: Same as first input
  Relational Operator : RelationalOperator  Operator=~=  InputSameDT=on
  Switch : Switch  Threshold=0.5  SaturateOnIntegerOverflow=on  InputSameDT=on
  Switch1 : Switch  Threshold=0.5  SaturateOnIntegerOverflow=on  InputSameDT=on
  Out : Outport
  wires:
  mult/1 -> Product/1
  Product/1 -> Switch/1
  Relational Operator/1 -> Switch/2, Switch1/2
  init/1 -> Switch/3
  Switch/1 -> Out/1
  div/1 -> Switch1/1, Relational Operator/1
  Switch1/1 -> Product/2
  Constant2/1 -> Relational Operator/2
  Constant1/1 -> Switch1/3

== system lib_all/SubSystem660/SubSystem686/SubSystem688/SubSystem691/SubSystem692 ==
  note: ***編集禁止***
  In1 : Inport
  In2 : Inport  Port=2
  RO1 : RelationalOperator  Operator=>  InputSameDT=on
  single1 : DataTypeConversion  OutDataTypeStr=single  SaturateOnIntegerOverflow=on  RndMeth=Zero
  single2 : DataTypeConversion  OutDataTypeStr=single  SaturateOnIntegerOverflow=on  RndMeth=Zero
  Out1 : Outport
  wires:
  RO1/1 -> Out1/1
  In2/1 -> single2/1
  In1/1 -> single1/1
  single1/1 -> RO1/1
  single2/1 -> RO1/2

== system lib_all/SubSystem660/SubSystem686/SubSystem688/SubSystem691/SubSystem693 ==
  note: ***編集禁止***
  In1 : Inport
  In2 : Inport  Port=2
  LO1 : Logic
  RO1 : RelationalOperator  Operator=<  InputSameDT=on
  RO2 : RelationalOperator  Operator=~=  InputSameDT=on
  single1 : DataTypeConversion  OutDataTypeStr=single  SaturateOnIntegerOverflow=on  RndMeth=Zero
  single2 : DataTypeConversion  OutDataTypeStr=single  SaturateOnIntegerOverflow=on  RndMeth=Zero
  Out1 : Outport
  wires:
  In2/1 -> single2/1
  In1/1 -> single1/1
  single1/1 -> RO1/1, RO2/1
  single2/1 -> RO1/2, RO2/2
  RO1/1 -> LO1/1
  RO2/1 -> LO1/2
  LO1/1 -> Out1/1

== system lib_all/SubSystem660/SubSystem686/SubSystem694/SubSystem698/SubSystem699 ==
  Block1612 : Inport
  Block1613 : Inport  Port=2
  Block1614 : MultiPortSwitch  zeroidx=off  RndMeth=Zero  SaturateOnIntegerOverflow=on  InputSameDT=on
  SubSystem700 : Subsystem  TreatAsAtomicUnit=on  mask(***ATC.DSRVEC***){bd_version=1.01.A, r0=DSM18, r1=-1, r2=, r3=, r4=, r5=, r6=, r7=, r8=, r9=, c0=, c1=, c2=, c3=, c4=, c5=, c6=, c7=, c8=, c9=}
      mask prompts: bd_version "Version"
      mask help: これはAutoCからのブロックです．編集禁止．
      settings: OpenFcn=warndlg('変更禁止!');
      mask Help: web(ui_blockhelpmng(gcb));
      mask Display: disp('ATC.DSRVEC')
  SubSystem701 : Subsystem  TreatAsAtomicUnit=on  mask(***ATC.DSRVEC***){bd_version=1.01.A, r0=DSM8, r1=-1, r2=, r3=, r4=, r5=, r6=, r7=, r8=, r9=, c0=, c1=, c2=, c3=, c4=, c5=, c6=, c7=, c8=, c9=}
      mask prompts: bd_version "Version"
      mask help: これはAutoCからのブロックです．編集禁止．
      settings: OpenFcn=warndlg('変更禁止!');
      mask Help: web(ui_blockhelpmng(gcb));
      mask Display: disp('ATC.DSRVEC')
  SubSystem702 : Subsystem  TreatAsAtomicUnit=on  mask(***ATC.DSRVEC***){bd_version=1.01.A, r0=DSM11, r1=-1, r2=, r3=, r4=, r5=, r6=, r7=, r8=, r9=, c0=, c1=, c2=, c3=, c4=, c5=, c6=, c7=, c8=, c9=}
      mask prompts: bd_version "Version"
      mask help: これはAutoCからのブロックです．編集禁止．
      settings: OpenFcn=warndlg('変更禁止!');
      mask Help: web(ui_blockhelpmng(gcb));
      mask Display: disp('ATC.DSRVEC')
  Block1615 : Outport
  wires:
  Block1613/1 -> SubSystem702/1(element), SubSystem701/1(element), SubSystem700/1(element)
  SubSystem702/1(Output) -> Block1614/4
  SubSystem701/1(Output) -> Block1614/3
  SubSystem700/1(Output) -> Block1614/2
  Block1614/1 -> Block1615/1
  Block1612/1 -> Block1614/1

== system lib_all/SubSystem660/SubSystem686/SubSystem694/SubSystem698/SubSystem703 ==
  Block1616 : Inport
  Block1617 : Inport  Port=2
  Block1618 : MultiPortSwitch  zeroidx=off  RndMeth=Zero  SaturateOnIntegerOverflow=on  InputSameDT=on
  SubSystem704 : Subsystem  TreatAsAtomicUnit=on  mask(***ATC.DSRVEC***){bd_version=1.01.A, r0=DSM18, r1=-1, r2=, r3=, r4=, r5=, r6=, r7=, r8=, r9=, c0=, c1=, c2=, c3=, c4=, c5=, c6=, c7=, c8=, c9=}
      mask prompts: bd_version "Version"
      mask help: これはAutoCからのブロックです．編集禁止．
      settings: OpenFcn=warndlg('変更禁止!');
      mask Help: web(ui_blockhelpmng(gcb));
      mask Display: disp('ATC.DSRVEC')
  SubSystem705 : Subsystem  TreatAsAtomicUnit=on  mask(***ATC.DSRVEC***){bd_version=1.01.A, r0=DSM8, r1=-1, r2=, r3=, r4=, r5=, r6=, r7=, r8=, r9=, c0=, c1=, c2=, c3=, c4=, c5=, c6=, c7=, c8=, c9=}
      mask prompts: bd_version "Version"
      mask help: これはAutoCからのブロックです．編集禁止．
      settings: OpenFcn=warndlg('変更禁止!');
      mask Help: web(ui_blockhelpmng(gcb));
      mask Display: disp('ATC.DSRVEC')
  SubSystem706 : Subsystem  TreatAsAtomicUnit=on  mask(***ATC.DSRVEC***){bd_version=1.01.A, r0=DSM11, r1=-1, r2=, r3=, r4=, r5=, r6=, r7=, r8=, r9=, c0=, c1=, c2=, c3=, c4=, c5=, c6=, c7=, c8=, c9=}
      mask prompts: bd_version "Version"
      mask help: これはAutoCからのブロックです．編集禁止．
      settings: OpenFcn=warndlg('変更禁止!');
      mask Help: web(ui_blockhelpmng(gcb));
      mask Display: disp('ATC.DSRVEC')
  Block1619 : Outport
  wires:
  Block1617/1 -> SubSystem706/1(element), SubSystem705/1(element), SubSystem704/1(element)
  SubSystem706/1(Output) -> Block1618/4
  SubSystem705/1(Output) -> Block1618/3
  SubSystem704/1(Output) -> Block1618/2
  Block1618/1 -> Block1619/1
  Block1616/1 -> Block1618/1

== system lib_all/SubSystem660/SubSystem686/SubSystem694/SubSystem707/SubSystem708 ==
  note: ***編集禁止***
  element : Inport
  Inside_DSR : DataStoreRead  DataStoreName=DSM4
  Selector : Selector  IndexOptions=Index vector (port)  Indices=[1 3]  OutputSizes=1
  Output : Outport
  wires:
  Selector/1 -> Output/1
  element/1 -> Selector/2
  Inside_DSR/1 -> Selector/1

== system lib_all/SubSystem660/SubSystem686/SubSystem694/SubSystem707/SubSystem709 ==
  Block1627 : Inport
  Block1628 : Inport  Port=2
  Block1629 : MultiPortSwitch  zeroidx=off  RndMeth=Zero  SaturateOnIntegerOverflow=on  InputSameDT=on
  SubSystem710 : Subsystem  TreatAsAtomicUnit=on  mask(***ATC.DSRVEC***){bd_version=1.01.A, r0=DSM18, r1=-1, r2=, r3=, r4=, r5=, r6=, r7=, r8=, r9=, c0=, c1=, c2=, c3=, c4=, c5=, c6=, c7=, c8=, c9=}
      mask prompts: bd_version "Version"
      mask help: これはAutoCからのブロックです．編集禁止．
      settings: OpenFcn=warndlg('変更禁止!');
      mask Help: web(ui_blockhelpmng(gcb));
      mask Display: disp('ATC.DSRVEC')
  SubSystem711 : Subsystem  TreatAsAtomicUnit=on  mask(***ATC.DSRVEC***){bd_version=1.01.A, r0=DSM8, r1=-1, r2=, r3=, r4=, r5=, r6=, r7=, r8=, r9=, c0=, c1=, c2=, c3=, c4=, c5=, c6=, c7=, c8=, c9=}
      mask prompts: bd_version "Version"
      mask help: これはAutoCからのブロックです．編集禁止．
      settings: OpenFcn=warndlg('変更禁止!');
      mask Help: web(ui_blockhelpmng(gcb));
      mask Display: disp('ATC.DSRVEC')
  SubSystem712 : Subsystem  TreatAsAtomicUnit=on  mask(***ATC.DSRVEC***){bd_version=1.01.A, r0=DSM11, r1=-1, r2=, r3=, r4=, r5=, r6=, r7=, r8=, r9=, c0=, c1=, c2=, c3=, c4=, c5=, c6=, c7=, c8=, c9=}
      mask prompts: bd_version "Version"
      mask help: これはAutoCからのブロックです．編集禁止．
      settings: OpenFcn=warndlg('変更禁止!');
      mask Help: web(ui_blockhelpmng(gcb));
      mask Display: disp('ATC.DSRVEC')
  Block1630 : Outport
  wires:
  Block1628/1 -> SubSystem712/1(element), SubSystem711/1(element), SubSystem710/1(element)
  SubSystem712/1(Output) -> Block1629/4
  SubSystem711/1(Output) -> Block1629/3
  SubSystem710/1(Output) -> Block1629/2
  Block1629/1 -> Block1630/1
  Block1627/1 -> Block1629/1

== system lib_all/SubSystem660/SubSystem686/SubSystem694/SubSystem707/SubSystem713 ==
  Block1631 : Inport
  Block1632 : Inport  Port=2
  Block1633 : MultiPortSwitch  zeroidx=off  RndMeth=Zero  SaturateOnIntegerOverflow=on  InputSameDT=on
  SubSystem714 : Subsystem  TreatAsAtomicUnit=on  mask(***ATC.DSRVEC***){bd_version=1.01.A, r0=DSM18, r1=-1, r2=, r3=, r4=, r5=, r6=, r7=, r8=, r9=, c0=, c1=, c2=, c3=, c4=, c5=, c6=, c7=, c8=, c9=}
      mask prompts: bd_version "Version"
      mask help: これはAutoCからのブロックです．編集禁止．
      settings: OpenFcn=warndlg('変更禁止!');
      mask Help: web(ui_blockhelpmng(gcb));
      mask Display: disp('ATC.DSRVEC')
  SubSystem715 : Subsystem  TreatAsAtomicUnit=on  mask(***ATC.DSRVEC***){bd_version=1.01.A, r0=DSM8, r1=-1, r2=, r3=, r4=, r5=, r6=, r7=, r8=, r9=, c0=, c1=, c2=, c3=, c4=, c5=, c6=, c7=, c8=, c9=}
      mask prompts: bd_version "Version"
      mask help: これはAutoCからのブロックです．編集禁止．
      settings: OpenFcn=warndlg('変更禁止!');
      mask Help: web(ui_blockhelpmng(gcb));
      mask Display: disp('ATC.DSRVEC')
  SubSystem716 : Subsystem  TreatAsAtomicUnit=on  mask(***ATC.DSRVEC***){bd_version=1.01.A, r0=DSM11, r1=-1, r2=, r3=, r4=, r5=, r6=, r7=, r8=, r9=, c0=, c1=, c2=, c3=, c4=, c5=, c6=, c7=, c8=, c9=}
      mask prompts: bd_version "Version"
      mask help: これはAutoCからのブロックです．編集禁止．
      settings: OpenFcn=warndlg('変更禁止!');
      mask Help: web(ui_blockhelpmng(gcb));
      mask Display: disp('ATC.DSRVEC')
  Block1634 : Outport
  wires:
  Block1632/1 -> SubSystem716/1(element), SubSystem715/1(element), SubSystem714/1(element)
  SubSystem716/1(Output) -> Block1633/4
  SubSystem715/1(Output) -> Block1633/3
  SubSystem714/1(Output) -> Block1633/2
  Block1633/1 -> Block1634/1
  Block1631/1 -> Block1633/1

== system lib_all/SubSystem660/SubSystem686/SubSystem694/SubSystem717/SubSystem718 ==
  note: ***編集禁止***
  element : Inport
  Inside_DSR : DataStoreRead  DataStoreName=DSM2
  Selector : Selector  IndexOptions=Index vector (port)  Indices=[1 3]  OutputSizes=1
  Output : Outport
  wires:
  Selector/1 -> Output/1
  element/1 -> Selector/2
  Inside_DSR/1 -> Selector/1

== system lib_all/SubSystem660/SubSystem686/SubSystem694/SubSystem717/SubSystem719 ==
  Block1642 : Inport
  Block1643 : Inport  Port=2
  Block1644 : MultiPortSwitch  zeroidx=off  RndMeth=Zero  SaturateOnIntegerOverflow=on  InputSameDT=on
  SubSystem720 : Subsystem  TreatAsAtomicUnit=on  mask(***ATC.DSRVEC***){bd_version=1.01.A, r0=DSM18, r1=-1, r2=, r3=, r4=, r5=, r6=, r7=, r8=, r9=, c0=, c1=, c2=, c3=, c4=, c5=, c6=, c7=, c8=, c9=}
      mask prompts: bd_version "Version"
      mask help: これはAutoCからのブロックです．編集禁止．
      settings: OpenFcn=warndlg('変更禁止!');
      mask Help: web(ui_blockhelpmng(gcb));
      mask Display: disp('ATC.DSRVEC')
  SubSystem721 : Subsystem  TreatAsAtomicUnit=on  mask(***ATC.DSRVEC***){bd_version=1.01.A, r0=DSM8, r1=-1, r2=, r3=, r4=, r5=, r6=, r7=, r8=, r9=, c0=, c1=, c2=, c3=, c4=, c5=, c6=, c7=, c8=, c9=}
      mask prompts: bd_version "Version"
      mask help: これはAutoCからのブロックです．編集禁止．
      settings: OpenFcn=warndlg('変更禁止!');
      mask Help: web(ui_blockhelpmng(gcb));
      mask Display: disp('ATC.DSRVEC')
  SubSystem722 : Subsystem  TreatAsAtomicUnit=on  mask(***ATC.DSRVEC***){bd_version=1.01.A, r0=DSM11, r1=-1, r2=, r3=, r4=, r5=, r6=, r7=, r8=, r9=, c0=, c1=, c2=, c3=, c4=, c5=, c6=, c7=, c8=, c9=}
      mask prompts: bd_version "Version"
      mask help: これはAutoCからのブロックです．編集禁止．
      settings: OpenFcn=warndlg('変更禁止!');
      mask Help: web(ui_blockhelpmng(gcb));
      mask Display: disp('ATC.DSRVEC')
  Block1645 : Outport
  wires:
  Block1643/1 -> SubSystem722/1(element), SubSystem721/1(element), SubSystem720/1(element)
  SubSystem722/1(Output) -> Block1644/4
  SubSystem721/1(Output) -> Block1644/3
  SubSystem720/1(Output) -> Block1644/2
  Block1644/1 -> Block1645/1
  Block1642/1 -> Block1644/1

== system lib_all/SubSystem660/SubSystem686/SubSystem694/SubSystem717/SubSystem723 ==
  Block1646 : Inport
  Block1647 : Inport  Port=2
  Block1648 : MultiPortSwitch  zeroidx=off  RndMeth=Zero  SaturateOnIntegerOverflow=on  InputSameDT=on
  SubSystem724 : Subsystem  TreatAsAtomicUnit=on  mask(***ATC.DSRVEC***){bd_version=1.01.A, r0=DSM18, r1=-1, r2=, r3=, r4=, r5=, r6=, r7=, r8=, r9=, c0=, c1=, c2=, c3=, c4=, c5=, c6=, c7=, c8=, c9=}
      mask prompts: bd_version "Version"
      mask help: これはAutoCからのブロックです．編集禁止．
      settings: OpenFcn=warndlg('変更禁止!');
      mask Help: web(ui_blockhelpmng(gcb));
      mask Display: disp('ATC.DSRVEC')
  SubSystem725 : Subsystem  TreatAsAtomicUnit=on  mask(***ATC.DSRVEC***){bd_version=1.01.A, r0=DSM8, r1=-1, r2=, r3=, r4=, r5=, r6=, r7=, r8=, r9=, c0=, c1=, c2=, c3=, c4=, c5=, c6=, c7=, c8=, c9=}
      mask prompts: bd_version "Version"
      mask help: これはAutoCからのブロックです．編集禁止．
      settings: OpenFcn=warndlg('変更禁止!');
      mask Help: web(ui_blockhelpmng(gcb));
      mask Display: disp('ATC.DSRVEC')
  SubSystem726 : Subsystem  TreatAsAtomicUnit=on  mask(***ATC.DSRVEC***){bd_version=1.01.A, r0=DSM11, r1=-1, r2=, r3=, r4=, r5=, r6=, r7=, r8=, r9=, c0=, c1=, c2=, c3=, c4=, c5=, c6=, c7=, c8=, c9=}
      mask prompts: bd_version "Version"
      mask help: これはAutoCからのブロックです．編集禁止．
      settings: OpenFcn=warndlg('変更禁止!');
      mask Help: web(ui_blockhelpmng(gcb));
      mask Display: disp('ATC.DSRVEC')
  Block1649 : Outport
  wires:
  Block1647/1 -> SubSystem726/1(element), SubSystem725/1(element), SubSystem724/1(element)
  SubSystem726/1(Output) -> Block1648/4
  SubSystem725/1(Output) -> Block1648/3
  SubSystem724/1(Output) -> Block1648/2
  Block1648/1 -> Block1649/1
  Block1646/1 -> Block1648/1

== system lib_all/SubSystem7/SubSystem134/SubSystem136/SubSystem139/SubSystem140 ==
  note: ***編集禁止***
  In1 : Inport
  In2 : Inport  Port=2
  RO1 : RelationalOperator  Operator=<=  InputSameDT=on
  single1 : DataTypeConversion  OutDataTypeStr=single  SaturateOnIntegerOverflow=on  RndMeth=Zero
  single2 : DataTypeConversion  OutDataTypeStr=single  SaturateOnIntegerOverflow=on  RndMeth=Zero
  Out1 : Outport
  wires:
  RO1/1 -> Out1/1
  In2/1 -> single2/1
  In1/1 -> single1/1
  single1/1 -> RO1/1
  single2/1 -> RO1/2

== system lib_all/SubSystem7/SubSystem134/SubSystem136/SubSystem139/SubSystem141 ==
  note: ***編集禁止***
  input : Inport
  element : Inport  Port=2
  Assignment : Assignment  IndexOptions=Index vector (port)  Indices=1  OutputSizes=[1 1]
  Inside_DSR : DataStoreRead  DataStoreName=DSM371
  Inside_DSW : DataStoreWrite  DataStoreName=DSM371
  wires:
  element/1 -> Assignment/3
  input/1 -> Assignment/2
  Inside_DSR/1 -> Assignment/1
  Assignment/1 -> Inside_DSW/1

== system lib_all/SubSystem7/SubSystem134/SubSystem136/SubSystem139/SubSystem142 ==
  note: ***編集禁止***
  input : Inport
  element : Inport  Port=2
  Assignment : Assignment  IndexOptions=Index vector (port)  Indices=1  OutputSizes=[1 1]
  Inside_DSR : DataStoreRead  DataStoreName=DSM373
  Inside_DSW : DataStoreWrite  DataStoreName=DSM373
  wires:
  element/1 -> Assignment/3
  input/1 -> Assignment/2
  Inside_DSR/1 -> Assignment/1
  Assignment/1 -> Inside_DSW/1

== system lib_all/SubSystem7/SubSystem134/SubSystem136/SubSystem139/SubSystem143 ==
  note: ***編集禁止***
  input : Inport
  element : Inport  Port=2
  Assignment : Assignment  IndexOptions=Index vector (port)  Indices=1  OutputSizes=[1 1]
  Inside_DSR : DataStoreRead  DataStoreName=DSM375
  Inside_DSW : DataStoreWrite  DataStoreName=DSM375
  wires:
  element/1 -> Assignment/3
  input/1 -> Assignment/2
  Inside_DSR/1 -> Assignment/1
  Assignment/1 -> Inside_DSW/1

== system lib_all/SubSystem7/SubSystem134/SubSystem136/SubSystem139/SubSystem144 ==
  Block333 : EnablePort
  Block334 : Constant  Value=Parameter131  OutDataTypeStr=double  SampleTime=-1
  Block335 : Constant  Value=10  OutDataTypeStr=double  SampleTime=-1
  Block336 : DataStoreWrite  DataStoreName=DSM365
  SubSystem145 : Subsystem  TreatAsAtomicUnit=on
  SubSystem147 : Subsystem  TreatAsAtomicUnit=on
      settings: RTWSystemCode=Inline
  wires:
  Block335/1 -> Block336/1
  Block334/1 -> SubSystem147/1(N)
  SubSystem147/1(action) -> SubSystem145/Trigger
  SubSystem147/2(i) -> SubSystem145/1(Block337)
